simulink model "ClimaCmftStMgr"  (R2022b)
settings: Solver=FixedStepDiscrete  StartTime=0.0  StopTime=10.0  FixedStep=0.1  RelTol=1e-3
(part 2 of 2)

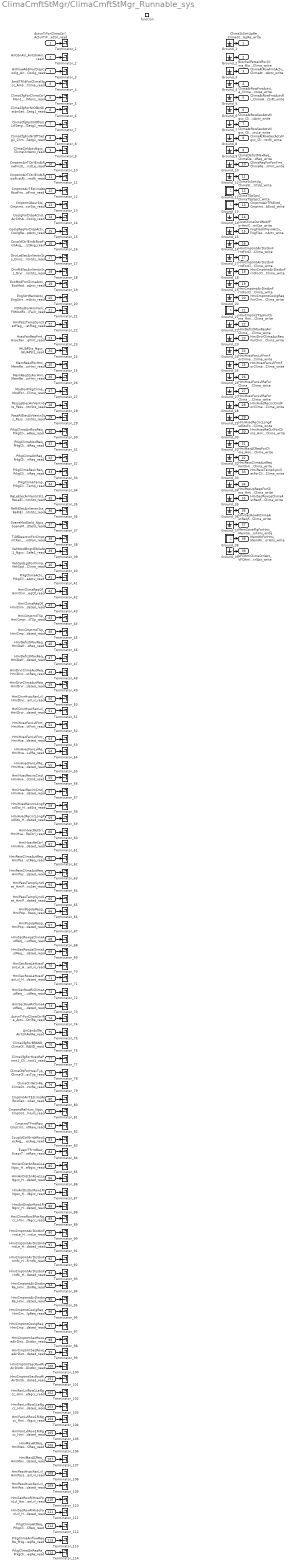
== system ClimaCmftStMgr/ClimaCmftStMgr_Runnable_sys ==
  ActvnTiForClimaCtrl_ActvnTiForClimaCtrl_read : Inport
  AirCdnAvl_AirCdnAvl_read : Inport  Port=2
  AirFlowAddlForDispCoolg_AirFlowAddlForDispCoolg_read : Inport  Port=3
  AmbTFildForClimaNgcc_AmbTFildForClima_read : Inport  Port=4
  ClimaCfgForClimaCtrlMan1_ClimaCfgForClimaCtrlMan1_read : Inport  Port=5
  ClimaCfgForNrOfAirDistbnSetg1_ClimaCfgForNrOfAirDistbnSetg1_read : Inport  Port=6
  ClimaCfgForNrOfFanLvlSetg1_ClimaCfgForNrOfFanLvlSetg1_read : Inport  Port=7
  ClimaCfgForNrOfTSetg1_ClimaCfgForNrOfTSetg1_read : Inport  Port=8
  ClimaCtrlActvNgcc_ClimaCtrlActv_read : Inport  Port=9
  CmptmtAirTCtrlErrAtRowFirstLe_CmptmtAirTCtrlErrAtRowFirstLe_read : Inport  Port=10
  CmptmtAirTCtrlErrAtRowFirstRi_CmptmtAirTCtrlErrAtRowFirstRi_read : Inport  Port=11
  CmptmtAirTEstimdAtRowFirst_CmptmtAirTEstimdAtRowFirst_read : Inport  Port=12
  CmptmtObsvrSts_CmptmtObsvrSts_read : Inport  Port=13
  CoolgForDispActvd_AirCdnActvdForDispCoolg_read : Inport  Port=14
  CoolgReqForDispActv_CoolgReqForDispActv_read : Inport  Port=15
  CoupldCtrlErrAtRowFirstAvg_CoupldCtrlErrAtRowFirstAvg_read : Inport  Port=16
  DrvrLeElecAirVentnSts_DrvrLeElecAirVentnSts_read : Inport  Port=17
  DrvrRiElecAirVentnSts_DrvrRiElecAirVentnSts_read : Inport  Port=18
  EcoModForClimaActv_EcoModForClimaActv_read : Inport  Port=19
  EngStrtRemActv_EngStrtRemActv_read : Inport  Port=20
  FltModForHmiFailr_FltModForHmiFailr_read : Inport  Port=21
  HmiPassTempSyncSetFlag_HmiPassTempSyncSetFlag_read : Inport  Port=22
  HvacFanReqFrnt_HvacFanReqFrnt_read : Inport  Port=23
  IHUAPSts_Ngcc_IHUAPSts_read : Inport  Port=24
  MemReadForHmi_MemReadForHmi_read : Inport  Port=25
  MemReqStsForHmi_MemReqStsForHmi_read : Inport  Port=26
  ModForPrkgClima_ModForPrkgClima_read : Inport  Port=27
  PassLeElecAirVentnSts_PassLeElecAirVentnSts_read : Inport  Port=28
  PassRiElecAirVentnSts_PassRiElecAirVentnSts_read : Inport  Port=29
  PrkgClimaAirflowReq_PrkgClimaAirflowReq_read : Inport  Port=30
  PrkgClimaModReq_PrkgClimaModReq_read : Inport  Port=31
  PrkgClimaOnReq_PrkgClimaOnReq_read : Inport  Port=32
  PrkgClimaRecirReq_PrkgClimaRecirReq_read : Inport  Port=33
  PrkgClimaTemp_PrkgClimaTemp_read : Inport  Port=34
  ReLeElecAirVentnSts_ReLeElecAirVentnSts_read : Inport  Port=35
  ReRiElecAirVentnSts_ReRiElecAirVentnSts_read : Inport  Port=36
  SceneModSeld_Ngcc_SceneModSeld_read : Inport  Port=37
  TiOfDeactvnForCmptmtFan_TiOfDeactvnForCmptmtFan_read : Inport  Port=38
  VehModMngtGlbSafe1_Ngcc_VehModMngtGlbSafe1_read : Inport  Port=39
  VehSpdLgtForClima_VehSpdLgtForClima_read : Inport  Port=40
  PrkgClimaActv_PrkgClimaActv_read : Inport  Port=41
  HmiClimaReqQf_HmiClimaReqQf_read : Inport  Port=42
  HmiClimaReqQf_HmiClimaReqQf_IsUpdated_read : Inport  Port=43
  HmiCmptmtTSp_HmiCmptmtTSp_read : Inport  Port=44
  HmiCmptmtTSp_HmiCmptmtTSp_IsUpdated_read : Inport  Port=45
  HmiDefrstMaxReq_HmiDefrstMaxReq_read : Inport  Port=46
  HmiDefrstMaxReq_HmiDefrstMaxReq_IsUpdated_read : Inport  Port=47
  HmiDrvrClimaAutReq_HmiDrvrClimaAutReq_read : Inport  Port=48
  HmiDrvrClimaAutReq_HmiDrvrClimaAutReq_IsUpdated_read : Inport  Port=49
  HmiDrvrHvacFanLvl_HmiDrvrHvacFanLvl_read : Inport  Port=50
  HmiDrvrHvacFanLvl_HmiDrvrHvacFanLvl_IsUpdated_read : Inport  Port=51
  HmiHvacFanLvlFrnt_HmiHvacFanLvlFrnt_read : Inport  Port=52
  HmiHvacFanLvlFrnt_HmiHvacFanLvlFrnt_IsUpdated_read : Inport  Port=53
  HmiHvacFanLvlRe_HmiHvacFanLvlRe_read : Inport  Port=54
  HmiHvacFanLvlRe_HmiHvacFanLvlRe_IsUpdated_read : Inport  Port=55
  HmiHvacRecircCmd_HmiHvacRecircCmd_read : Inport  Port=56
  HmiHvacRecircCmd_HmiHvacRecircCmd_IsUpdated_read : Inport  Port=57
  HmiHvacRecircLongPsdSts_HmiHvacRecircLongPsdSts_read : Inport  Port=58
  HmiHvacRecircLongPsdSts_HmiHvacRecircLongPsdSts_IsUpdated_read : Inport  Port=59
  HmiHvacReCtrl_HmiHvacReCtrl_read : Inport  Port=60
  HmiHvacReCtrl_HmiHvacReCtrl_IsUpdated_read : Inport  Port=61
  HmiPassClimaAutReq_HmiPassClimaAutReq_read : Inport  Port=62
  HmiPassClimaAutReq_HmiPassClimaAutReq_IsUpdated_read : Inport  Port=63
  HmiPassTempSyncSet_HmiPassTempSyncSet_read : Inport  Port=64
  HmiPassTempSyncSet_HmiPassTempSyncSet_IsUpdated_read : Inport  Port=65
  HmiPopUpResp_HmiPopUpResp_read : Inport  Port=66
  HmiPopUpResp_HmiPopUpResp_IsUpdated_read : Inport  Port=67
  HmiSecRowLeClimaAutReq_HmiSecRowLeClimaAutReq_read : Inport  Port=68
  HmiSecRowLeClimaAutReq_HmiSecRowLeClimaAutReq_IsUpdated_read : Inport  Port=69
  HmiSecRowLeHvacFanLvl_HmiSecRowLeHvacFanLvl_read : Inport  Port=70
  HmiSecRowLeHvacFanLvl_HmiSecRowLeHvacFanLvl_IsUpdated_read : Inport  Port=71
  HmiSecRowRiClimaAutReq_HmiSecRowRiClimaAutReq_read : Inport  Port=72
  HmiSecRowRiClimaAutReq_HmiSecRowRiClimaAutReq_IsUpdated_read : Inport  Port=73
  ActvnTiForClimaCtrlRe_ActvnTiForClimaCtrlRe_read : Inport  Port=74
  AirCdnAvlRe_AirCdnAvlRe_read : Inport  Port=75
  ClimaCfgForBRAND_ClimaCfgForBRAND_read : Inport  Port=76
  ClimaCfgForHvacRePrsnt1_ClimaCfgForHvacRePrsnt1_read : Inport  Port=77
  ClimaCfgForHvacTyp_ClimaCfgForHvacTyp_read : Inport  Port=78
  ClimaCtrlActvRe_ClimaCtrlActvRe_read : Inport  Port=79
  CmptmtAirTEstimdAtRowSec_CmptmtAirTEstimdAtRowSec_read : Inport  Port=80
  CmptmtRelHum_Ngcc_CmptmtRelHum_read : Inport  Port=81
  CmptmtTFrntRaw_CmptmtTFrntRaw_read : Inport  Port=82
  CoupldCtrlErrAtRowSecAvg_CoupldCtrlErrAtRowSecAvg_read : Inport  Port=83
  EvaprTFrntRaw_EvaprTFrntRaw_read : Inport  Port=84
  HmiAirDistbnRow1LeNgcc_HmiAirDistbnRow1LeNgcc_read : Inport  Port=85
  HmiAirDistbnRow1LeNgcc_HmiAirDistbnRow1LeNgcc_IsUpdated_read : Inport  Port=86
  HmiAirDistbnRow1RiNgcc_HmiAirDistbnRow1RiNgcc_read : Inport  Port=87
  HmiAirDistbnRow1RiNgcc_HmiAirDistbnRow1RiNgcc_IsUpdated_read : Inport  Port=88
  HmiClimaRow3PwrNgcc_HmiClimaRow3PwrNgcc_read : Inport  Port=89
  HmiCmptmtAirDistbnFrntLe_HmiCmptmtAirDistbnFrntLe_read : Inport  Port=90
  HmiCmptmtAirDistbnFrntLe_HmiCmptmtAirDistbnFrntLe_IsUpdated_read : Inport  Port=91
  HmiCmptmtAirDistbnFrntRi_HmiCmptmtAirDistbnFrntRi_read : Inport  Port=92
  HmiCmptmtAirDistbnFrntRi_HmiCmptmtAirDistbnFrntRi_IsUpdated_read : Inport  Port=93
  HmiCmptmtAirDistbnRe_HmiCmptmtAirDistbnRe_read : Inport  Port=94
  HmiCmptmtAirDistbnRe_HmiCmptmtAirDistbnRe_IsUpdated_read : Inport  Port=95
  HmiCmptmtCoolgReq_HmiCmptmtCoolgReq_read : Inport  Port=96
  HmiCmptmtCoolgReq_HmiCmptmtCoolgReq_IsUpdated_read : Inport  Port=97
  HmiCmptmtSecRowLeAirDistbn_HmiCmptmtSecRowLeAirDistbn_read : Inport  Port=98
  HmiCmptmtSecRowLeAirDistbn_HmiCmptmtSecRowLeAirDistbn_IsUpdated_read : Inport  Port=99
  HmiCmptmtSecRowRiAirDistbn_HmiCmptmtSecRowRiAirDistbn_read : Inport  Port=100
  HmiCmptmtSecRowRiAirDistbn_HmiCmptmtSecRowRiAirDistbn_IsUpdated_read : Inport  Port=101
  HmiFanLvlRow1LeNgcc_HmiFanLvlRow1LeNgcc_read : Inport  Port=102
  HmiFanLvlRow1LeNgcc_HmiFanLvlRow1LeNgcc_IsUpdated_read : Inport  Port=103
  HmiFanLvlRow1RiNgcc_HmiFanLvlRow1RiNgcc_read : Inport  Port=104
  HmiFanLvlRow1RiNgcc_HmiFanLvlRow1RiNgcc_IsUpdated_read : Inport  Port=105
  HmiMaxACReq_HmiMaxACReq_read : Inport  Port=106
  HmiMaxACReq_HmiMaxACReq_IsUpdated_read : Inport  Port=107
  HmiPassHvacFanLvl_HmiPassHvacFanLvl_read : Inport  Port=108
  HmiPassHvacFanLvl_HmiPassHvacFanLvl_IsUpdated_read : Inport  Port=109
  HmiSecRowRiHvacFanLvl_HmiSecRowRiHvacFanLvl_read : Inport  Port=110
  HmiSecRowRiHvacFanLvl_HmiSecRowRiHvacFanLvl_IsUpdated_read : Inport  Port=111
  PrkgClimaACReq_PrkgClimaACReq_read : Inport  Port=112
  PrkgClimaAirflowReqRe_PrkgClimaAirflowReqRe_read : Inport  Port=113
  PrkgClimaOnReqRe_PrkgClimaOnReqRe_read : Inport  Port=114
  function : TriggerPort  TriggerType=function-call  VariantControl=(inherit)  SampleTimeType=periodic  SampleTime=-1
  Ground_{1..11,14..20,22..37,39} (35 alike) : Ground
  Ground_12 : Subsystem
  Ground_13 : Subsystem
  Ground_21 : Subsystem
  Ground_38 : Subsystem
  Terminator_{1..114} (114 alike) : Terminator
  ClimaStStrtUpRe_ClimaStStrtUpRe_write : Outport
      settings: EnsureOutportIsVirtual=on
  BiochalPatseldForClima_BiochalPatseldForClima_write : Outport  Port=2
      settings: EnsureOutportIsVirtual=on
  ClimaAtRowFrntActv_ClimaAtRowFrntActv_write : Outport  Port=3
      settings: EnsureOutportIsVirtual=on
  ClimaAtRowFrntActvLe_ClimaAtRowFrntActvLe_write : Outport  Port=4
      settings: EnsureOutportIsVirtual=on
  ClimaAtRowFrntActvRi_ClimaAtRowFrntActvRi_write : Outport  Port=5
      settings: EnsureOutportIsVirtual=on
  ClimaAtRowSecActvNgcc_ClimaAtRowSecActv_write : Outport  Port=6
      settings: EnsureOutportIsVirtual=on
  ClimaAtRowSecActvNgcc_ClimaAtRowSecActvLe_write : Outport  Port=7
      settings: EnsureOutportIsVirtual=on
  ClimaAtRowSecActvNgcc_ClimaAtRowSecActvRi_write : Outport  Port=8
      settings: EnsureOutportIsVirtual=on
  ClimaDefrstMaxReq_ClimaDefrstMaxReq_write : Outport  Port=9
      settings: EnsureOutportIsVirtual=on
  ClimaReqForFanFrnt_ClimaReqForFanFrnt_write : Outport  Port=10
      settings: EnsureOutportIsVirtual=on
  ClimaStStrtUp_ClimaStStrtUp_write : Outport  Port=11
      settings: EnsureOutportIsVirtual=on
  ClimaTSpSpcl_ClimaTSpSpcl_write : Outport  Port=12
      settings: EnsureOutportIsVirtual=on
  CmptmtAirTFbExtd_CmptmtAirTFbExtd_write : Outport  Port=13
      settings: EnsureOutportIsVirtual=on
  CustClimaOvrdNotifForHmiCpt_CustClimaOvrdNotifForHmiCpt_write : Outport  Port=14
      settings: EnsureOutportIsVirtual=on
  FogFlashPrevnActv_FogFlashPrevnActv_write : Outport  Port=15
      settings: EnsureOutportIsVirtual=on
  HmiCmptmtAirDistbnFrntForClima_HmiCmptmtAirDistbnFrntForClima_write : Outport  Port=16
      settings: EnsureOutportIsVirtual=on
  HmiCmptmtAirDistbnFrntForClima_HmiCmptmtAirDistbnFrntRiForClima_write : Outport  Port=17
      settings: EnsureOutportIsVirtual=on
  HmiCmptmtAirDistbnFrntForClima_HmiCmptmtAirDistbnReForClima_write : Outport  Port=18
      settings: EnsureOutportIsVirtual=on
  HmiCmptmtAirDistbnFrntForClima_HmiCmptmtAirDistbnReRiForClima_write : Outport  Port=19
      settings: EnsureOutportIsVirtual=on
  HmiCmptmtCoolgReqForClima_HmiCmptmtCoolgReqForClima_write : Outport  Port=20
      settings: EnsureOutportIsVirtual=on
  HmiCmptmtTSpForClima_HmiCmptmtTSpForClima_write : Outport  Port=21
      settings: EnsureOutportIsVirtual=on
  HmiDefrstMaxReqForClima_HmiDefrstMaxReqForClima_write : Outport  Port=22
      settings: EnsureOutportIsVirtual=on
  HmiDrvrClimaAutReqForClima_HmiDrvrClimaAutReqForClima_write : Outport  Port=23
      settings: EnsureOutportIsVirtual=on
  HmiHvacFanLvlFrntForClima_HmiHvacFanLvlFrntForClima_write : Outport  Port=24
      settings: EnsureOutportIsVirtual=on
  HmiHvacFanLvlFrntForClima_HmiHvacFanLvlFrntRiForClima_write : Outport  Port=25
      settings: EnsureOutportIsVirtual=on
  HmiHvacFanLvlReForClima_HmiHvacFanLvlReForClima_write : Outport  Port=26
      settings: EnsureOutportIsVirtual=on
  HmiHvacFanLvlReForClima_HmiHvacFanLvlReRiForClima_write : Outport  Port=27
      settings: EnsureOutportIsVirtual=on
  HmiHvacRecircCmdForClima_HmiHvacRecircCmdForClima_write : Outport  Port=28
      settings: EnsureOutportIsVirtual=on
  HmiHvacRecircLongPsdStsForClima_HmiHvacRecircLongPsdStsForClima_write : Outport  Port=29
      settings: EnsureOutportIsVirtual=on
  HmiHvacReCtrlForClima_HmiHvacReCtrlForClima_write : Outport  Port=30
      settings: EnsureOutportIsVirtual=on
  HmiMaxACReqForClima_HmiMaxACReqForClima_write : Outport  Port=31
      settings: EnsureOutportIsVirtual=on
  HmiPassClimaAutReqForClima_HmiPassClimaAutReqForClima_write : Outport  Port=32
      settings: EnsureOutportIsVirtual=on
  HmiPassTempSyncSetForClima_HmiPassTempSyncSetForClima_write : Outport  Port=33
      settings: EnsureOutportIsVirtual=on
  HmiPopUpRespForClima_HmiPopUpRespForClima_write : Outport  Port=34
      settings: EnsureOutportIsVirtual=on
  HmiSecRowLeClimaAutReqForClima_HmiSecRowLeClimaAutReqForClima_write : Outport  Port=35
      settings: EnsureOutportIsVirtual=on
  HmiSecRowRiClimaAutReqForClima_HmiSecRowRiClimaAutReqForClima_write : Outport  Port=36
      settings: EnsureOutportIsVirtual=on
  MemSaveFlgForHmi_MemSaveFlgForHmi_write : Outport  Port=37
      settings: EnsureOutportIsVirtual=on
  MemWrForHmi_MemWrForHmi_write : Outport  Port=38
      settings: EnsureOutportIsVirtual=on
  VFCHmiClimaCtrlAct_VFCHmiClimaCtrlAct_write : Outport  Port=39
      settings: EnsureOutportIsVirtual=on
  wires:
  ActvnTiForClimaCtrl_ActvnTiForClimaCtrl_read/1 -> Terminator_1/1
  AirCdnAvl_AirCdnAvl_read/1 -> Terminator_2/1
  AirFlowAddlForDispCoolg_AirFlowAddlForDispCoolg_read/1 -> Terminator_3/1
  AmbTFildForClimaNgcc_AmbTFildForClima_read/1 -> Terminator_4/1
  ClimaCfgForClimaCtrlMan1_ClimaCfgForClimaCtrlMan1_read/1 -> Terminator_5/1
  ClimaCfgForNrOfAirDistbnSetg1_ClimaCfgForNrOfAirDistbnSetg1_read/1 -> Terminator_6/1
  ClimaCfgForNrOfFanLvlSetg1_ClimaCfgForNrOfFanLvlSetg1_read/1 -> Terminator_7/1
  ClimaCfgForNrOfTSetg1_ClimaCfgForNrOfTSetg1_read/1 -> Terminator_8/1
  ClimaCtrlActvNgcc_ClimaCtrlActv_read/1 -> Terminator_9/1
  CmptmtAirTCtrlErrAtRowFirstLe_CmptmtAirTCtrlErrAtRowFirstLe_read/1 -> Terminator_10/1
  CmptmtAirTCtrlErrAtRowFirstRi_CmptmtAirTCtrlErrAtRowFirstRi_read/1 -> Terminator_11/1
  CmptmtAirTEstimdAtRowFirst_CmptmtAirTEstimdAtRowFirst_read/1 -> Terminator_12/1
  CmptmtObsvrSts_CmptmtObsvrSts_read/1 -> Terminator_13/1
  CoolgForDispActvd_AirCdnActvdForDispCoolg_read/1 -> Terminator_14/1
  CoolgReqForDispActv_CoolgReqForDispActv_read/1 -> Terminator_15/1
  CoupldCtrlErrAtRowFirstAvg_CoupldCtrlErrAtRowFirstAvg_read/1 -> Terminator_16/1
  DrvrLeElecAirVentnSts_DrvrLeElecAirVentnSts_read/1 -> Terminator_17/1
  DrvrRiElecAirVentnSts_DrvrRiElecAirVentnSts_read/1 -> Terminator_18/1
  EcoModForClimaActv_EcoModForClimaActv_read/1 -> Terminator_19/1
  EngStrtRemActv_EngStrtRemActv_read/1 -> Terminator_20/1
  FltModForHmiFailr_FltModForHmiFailr_read/1 -> Terminator_21/1
  HmiPassTempSyncSetFlag_HmiPassTempSyncSetFlag_read/1 -> Terminator_22/1
  HvacFanReqFrnt_HvacFanReqFrnt_read/1 -> Terminator_23/1
  IHUAPSts_Ngcc_IHUAPSts_read/1 -> Terminator_24/1
  MemReadForHmi_MemReadForHmi_read/1 -> Terminator_25/1
  MemReqStsForHmi_MemReqStsForHmi_read/1 -> Terminator_26/1
  ModForPrkgClima_ModForPrkgClima_read/1 -> Terminator_27/1
  PassLeElecAirVentnSts_PassLeElecAirVentnSts_read/1 -> Terminator_28/1
  PassRiElecAirVentnSts_PassRiElecAirVentnSts_read/1 -> Terminator_29/1
  PrkgClimaAirflowReq_PrkgClimaAirflowReq_read/1 -> Terminator_30/1
  PrkgClimaModReq_PrkgClimaModReq_read/1 -> Terminator_31/1
  PrkgClimaOnReq_PrkgClimaOnReq_read/1 -> Terminator_32/1
  PrkgClimaRecirReq_PrkgClimaRecirReq_read/1 -> Terminator_33/1
  PrkgClimaTemp_PrkgClimaTemp_read/1 -> Terminator_34/1
  ReLeElecAirVentnSts_ReLeElecAirVentnSts_read/1 -> Terminator_35/1
  ReRiElecAirVentnSts_ReRiElecAirVentnSts_read/1 -> Terminator_36/1
  SceneModSeld_Ngcc_SceneModSeld_read/1 -> Terminator_37/1
  TiOfDeactvnForCmptmtFan_TiOfDeactvnForCmptmtFan_read/1 -> Terminator_38/1
  VehModMngtGlbSafe1_Ngcc_VehModMngtGlbSafe1_read/1 -> Terminator_39/1
  VehSpdLgtForClima_VehSpdLgtForClima_read/1 -> Terminator_40/1
  PrkgClimaActv_PrkgClimaActv_read/1 -> Terminator_41/1
  HmiClimaReqQf_HmiClimaReqQf_read/1 -> Terminator_42/1
  HmiClimaReqQf_HmiClimaReqQf_IsUpdated_read/1 -> Terminator_43/1
  HmiCmptmtTSp_HmiCmptmtTSp_read/1 -> Terminator_44/1
  HmiCmptmtTSp_HmiCmptmtTSp_IsUpdated_read/1 -> Terminator_45/1
  HmiDefrstMaxReq_HmiDefrstMaxReq_read/1 -> Terminator_46/1
  HmiDefrstMaxReq_HmiDefrstMaxReq_IsUpdated_read/1 -> Terminator_47/1
  HmiDrvrClimaAutReq_HmiDrvrClimaAutReq_read/1 -> Terminator_48/1
  HmiDrvrClimaAutReq_HmiDrvrClimaAutReq_IsUpdated_read/1 -> Terminator_49/1
  HmiDrvrHvacFanLvl_HmiDrvrHvacFanLvl_read/1 -> Terminator_50/1
  HmiDrvrHvacFanLvl_HmiDrvrHvacFanLvl_IsUpdated_read/1 -> Terminator_51/1
  HmiHvacFanLvlFrnt_HmiHvacFanLvlFrnt_read/1 -> Terminator_52/1
  HmiHvacFanLvlFrnt_HmiHvacFanLvlFrnt_IsUpdated_read/1 -> Terminator_53/1
  HmiHvacFanLvlRe_HmiHvacFanLvlRe_read/1 -> Terminator_54/1
  HmiHvacFanLvlRe_HmiHvacFanLvlRe_IsUpdated_read/1 -> Terminator_55/1
  HmiHvacRecircCmd_HmiHvacRecircCmd_read/1 -> Terminator_56/1
  HmiHvacRecircCmd_HmiHvacRecircCmd_IsUpdated_read/1 -> Terminator_57/1
  HmiHvacRecircLongPsdSts_HmiHvacRecircLongPsdSts_read/1 -> Terminator_58/1
  HmiHvacRecircLongPsdSts_HmiHvacRecircLongPsdSts_IsUpdated_read/1 -> Terminator_59/1
  HmiHvacReCtrl_HmiHvacReCtrl_read/1 -> Terminator_60/1
  HmiHvacReCtrl_HmiHvacReCtrl_IsUpdated_read/1 -> Terminator_61/1
  HmiPassClimaAutReq_HmiPassClimaAutReq_read/1 -> Terminator_62/1
  HmiPassClimaAutReq_HmiPassClimaAutReq_IsUpdated_read/1 -> Terminator_63/1
  HmiPassTempSyncSet_HmiPassTempSyncSet_read/1 -> Terminator_64/1
  HmiPassTempSyncSet_HmiPassTempSyncSet_IsUpdated_read/1 -> Terminator_65/1
  HmiPopUpResp_HmiPopUpResp_read/1 -> Terminator_66/1
  HmiPopUpResp_HmiPopUpResp_IsUpdated_read/1 -> Terminator_67/1
  HmiSecRowLeClimaAutReq_HmiSecRowLeClimaAutReq_read/1 -> Terminator_68/1
  HmiSecRowLeClimaAutReq_HmiSecRowLeClimaAutReq_IsUpdated_read/1 -> Terminator_69/1
  HmiSecRowLeHvacFanLvl_HmiSecRowLeHvacFanLvl_read/1 -> Terminator_70/1
  HmiSecRowLeHvacFanLvl_HmiSecRowLeHvacFanLvl_IsUpdated_read/1 -> Terminator_71/1
  HmiSecRowRiClimaAutReq_HmiSecRowRiClimaAutReq_read/1 -> Terminator_72/1
  HmiSecRowRiClimaAutReq_HmiSecRowRiClimaAutReq_IsUpdated_read/1 -> Terminator_73/1
  ActvnTiForClimaCtrlRe_ActvnTiForClimaCtrlRe_read/1 -> Terminator_74/1
  AirCdnAvlRe_AirCdnAvlRe_read/1 -> Terminator_75/1
  ClimaCfgForBRAND_ClimaCfgForBRAND_read/1 -> Terminator_76/1
  ClimaCfgForHvacRePrsnt1_ClimaCfgForHvacRePrsnt1_read/1 -> Terminator_77/1
  ClimaCfgForHvacTyp_ClimaCfgForHvacTyp_read/1 -> Terminator_78/1
  ClimaCtrlActvRe_ClimaCtrlActvRe_read/1 -> Terminator_79/1
  CmptmtAirTEstimdAtRowSec_CmptmtAirTEstimdAtRowSec_read/1 -> Terminator_80/1
  CmptmtRelHum_Ngcc_CmptmtRelHum_read/1 -> Terminator_81/1
  CmptmtTFrntRaw_CmptmtTFrntRaw_read/1 -> Terminator_82/1
  CoupldCtrlErrAtRowSecAvg_CoupldCtrlErrAtRowSecAvg_read/1 -> Terminator_83/1
  EvaprTFrntRaw_EvaprTFrntRaw_read/1 -> Terminator_84/1
  HmiAirDistbnRow1LeNgcc_HmiAirDistbnRow1LeNgcc_read/1 -> Terminator_85/1
  HmiAirDistbnRow1LeNgcc_HmiAirDistbnRow1LeNgcc_IsUpdated_read/1 -> Terminator_86/1
  HmiAirDistbnRow1RiNgcc_HmiAirDistbnRow1RiNgcc_read/1 -> Terminator_87/1
  HmiAirDistbnRow1RiNgcc_HmiAirDistbnRow1RiNgcc_IsUpdated_read/1 -> Terminator_88/1
  HmiClimaRow3PwrNgcc_HmiClimaRow3PwrNgcc_read/1 -> Terminator_89/1
  HmiCmptmtAirDistbnFrntLe_HmiCmptmtAirDistbnFrntLe_read/1 -> Terminator_90/1
  HmiCmptmtAirDistbnFrntLe_HmiCmptmtAirDistbnFrntLe_IsUpdated_read/1 -> Terminator_91/1
  HmiCmptmtAirDistbnFrntRi_HmiCmptmtAirDistbnFrntRi_read/1 -> Terminator_92/1
  HmiCmptmtAirDistbnFrntRi_HmiCmptmtAirDistbnFrntRi_IsUpdated_read/1 -> Terminator_93/1
  HmiCmptmtAirDistbnRe_HmiCmptmtAirDistbnRe_read/1 -> Terminator_94/1
  HmiCmptmtAirDistbnRe_HmiCmptmtAirDistbnRe_IsUpdated_read/1 -> Terminator_95/1
  HmiCmptmtCoolgReq_HmiCmptmtCoolgReq_read/1 -> Terminator_96/1
  HmiCmptmtCoolgReq_HmiCmptmtCoolgReq_IsUpdated_read/1 -> Terminator_97/1
  HmiCmptmtSecRowLeAirDistbn_HmiCmptmtSecRowLeAirDistbn_read/1 -> Terminator_98/1
  HmiCmptmtSecRowLeAirDistbn_HmiCmptmtSecRowLeAirDistbn_IsUpdated_read/1 -> Terminator_99/1
  HmiCmptmtSecRowRiAirDistbn_HmiCmptmtSecRowRiAirDistbn_read/1 -> Terminator_100/1
  HmiCmptmtSecRowRiAirDistbn_HmiCmptmtSecRowRiAirDistbn_IsUpdated_read/1 -> Terminator_101/1
  HmiFanLvlRow1LeNgcc_HmiFanLvlRow1LeNgcc_read/1 -> Terminator_102/1
  HmiFanLvlRow1LeNgcc_HmiFanLvlRow1LeNgcc_IsUpdated_read/1 -> Terminator_103/1
  HmiFanLvlRow1RiNgcc_HmiFanLvlRow1RiNgcc_read/1 -> Terminator_104/1
  HmiFanLvlRow1RiNgcc_HmiFanLvlRow1RiNgcc_IsUpdated_read/1 -> Terminator_105/1
  HmiMaxACReq_HmiMaxACReq_read/1 -> Terminator_106/1
  HmiMaxACReq_HmiMaxACReq_IsUpdated_read/1 -> Terminator_107/1
  HmiPassHvacFanLvl_HmiPassHvacFanLvl_read/1 -> Terminator_108/1
  HmiPassHvacFanLvl_HmiPassHvacFanLvl_IsUpdated_read/1 -> Terminator_109/1
  HmiSecRowRiHvacFanLvl_HmiSecRowRiHvacFanLvl_read/1 -> Terminator_110/1
  HmiSecRowRiHvacFanLvl_HmiSecRowRiHvacFanLvl_IsUpdated_read/1 -> Terminator_111/1
  PrkgClimaACReq_PrkgClimaACReq_read/1 -> Terminator_112/1
  PrkgClimaAirflowReqRe_PrkgClimaAirflowReqRe_read/1 -> Terminator_113/1
  PrkgClimaOnReqRe_PrkgClimaOnReqRe_read/1 -> Terminator_114/1
  Ground_1/1 -> ClimaStStrtUpRe_ClimaStStrtUpRe_write/1
  Ground_2/1 -> BiochalPatseldForClima_BiochalPatseldForClima_write/1
  Ground_3/1 -> ClimaAtRowFrntActv_ClimaAtRowFrntActv_write/1
  Ground_4/1 -> ClimaAtRowFrntActvLe_ClimaAtRowFrntActvLe_write/1
  Ground_5/1 -> ClimaAtRowFrntActvRi_ClimaAtRowFrntActvRi_write/1
  Ground_6/1 -> ClimaAtRowSecActvNgcc_ClimaAtRowSecActv_write/1
  Ground_7/1 -> ClimaAtRowSecActvNgcc_ClimaAtRowSecActvLe_write/1
  Ground_8/1 -> ClimaAtRowSecActvNgcc_ClimaAtRowSecActvRi_write/1
  Ground_9/1 -> ClimaDefrstMaxReq_ClimaDefrstMaxReq_write/1
  Ground_10/1 -> ClimaReqForFanFrnt_ClimaReqForFanFrnt_write/1
  Ground_11/1 -> ClimaStStrtUp_ClimaStStrtUp_write/1
  Ground_12/1(Out1) -> ClimaTSpSpcl_ClimaTSpSpcl_write/1
  Ground_13/1(Out1) -> CmptmtAirTFbExtd_CmptmtAirTFbExtd_write/1
  Ground_14/1 -> CustClimaOvrdNotifForHmiCpt_CustClimaOvrdNotifForHmiCpt_write/1
  Ground_15/1 -> FogFlashPrevnActv_FogFlashPrevnActv_write/1
  Ground_16/1 -> HmiCmptmtAirDistbnFrntForClima_HmiCmptmtAirDistbnFrntForClima_write/1
  Ground_17/1 -> HmiCmptmtAirDistbnFrntForClima_HmiCmptmtAirDistbnFrntRiForClima_write/1
  Ground_18/1 -> HmiCmptmtAirDistbnFrntForClima_HmiCmptmtAirDistbnReForClima_write/1
  Ground_19/1 -> HmiCmptmtAirDistbnFrntForClima_HmiCmptmtAirDistbnReRiForClima_write/1
  Ground_20/1 -> HmiCmptmtCoolgReqForClima_HmiCmptmtCoolgReqForClima_write/1
  Ground_21/1(Out1) -> HmiCmptmtTSpForClima_HmiCmptmtTSpForClima_write/1
  Ground_22/1 -> HmiDefrstMaxReqForClima_HmiDefrstMaxReqForClima_write/1
  Ground_23/1 -> HmiDrvrClimaAutReqForClima_HmiDrvrClimaAutReqForClima_write/1
  Ground_24/1 -> HmiHvacFanLvlFrntForClima_HmiHvacFanLvlFrntForClima_write/1
  Ground_25/1 -> HmiHvacFanLvlFrntForClima_HmiHvacFanLvlFrntRiForClima_write/1
  Ground_26/1 -> HmiHvacFanLvlReForClima_HmiHvacFanLvlReForClima_write/1
  Ground_27/1 -> HmiHvacFanLvlReForClima_HmiHvacFanLvlReRiForClima_write/1
  Ground_28/1 -> HmiHvacRecircCmdForClima_HmiHvacRecircCmdForClima_write/1
  Ground_29/1 -> HmiHvacRecircLongPsdStsForClima_HmiHvacRecircLongPsdStsForClima_write/1
  Ground_30/1 -> HmiHvacReCtrlForClima_HmiHvacReCtrlForClima_write/1
  Ground_31/1 -> HmiMaxACReqForClima_HmiMaxACReqForClima_write/1
  Ground_32/1 -> HmiPassClimaAutReqForClima_HmiPassClimaAutReqForClima_write/1
  Ground_33/1 -> HmiPassTempSyncSetForClima_HmiPassTempSyncSetForClima_write/1
  Ground_34/1 -> HmiPopUpRespForClima_HmiPopUpRespForClima_write/1
  Ground_35/1 -> HmiSecRowLeClimaAutReqForClima_HmiSecRowLeClimaAutReqForClima_write/1
  Ground_36/1 -> HmiSecRowRiClimaAutReqForClima_HmiSecRowRiClimaAutReqForClima_write/1
  Ground_37/1 -> MemSaveFlgForHmi_MemSaveFlgForHmi_write/1
  Ground_38/1(Out1) -> MemWrForHmi_MemWrForHmi_write/1
  Ground_39/1 -> VFCHmiClimaCtrlAct_VFCHmiClimaCtrlAct_write/1

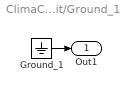
== system ClimaCmftStMgr/ClimaCmftStMgr_Init/Ground_1 ==
  Ground_1 : Ground
  Out1 : Outport  OutDataTypeStr=Bus: CmptmtAirTEstimdExtd  PortDimensions=1
  wires:
  Ground_1/1 -> Out1/1

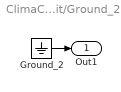
== system ClimaCmftStMgr/ClimaCmftStMgr_Init/Ground_2 ==
  Ground_2 : Ground
  Out1 : Outport  OutDataTypeStr=Bus: HmiCmptmtTSp  PortDimensions=1
  wires:
  Ground_2/1 -> Out1/1

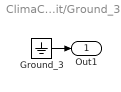
== system ClimaCmftStMgr/ClimaCmftStMgr_Init/Ground_3 ==
  Ground_3 : Ground
  Out1 : Outport  OutDataTypeStr=Bus: MemRecForHmi  PortDimensions=1
  wires:
  Ground_3/1 -> Out1/1

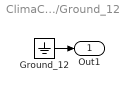
== system ClimaCmftStMgr/ClimaCmftStMgr_Runnable_sys/Ground_12 ==
  Ground_12 : Ground
  Out1 : Outport  OutDataTypeStr=Bus: ClimaTSpSpcl  PortDimensions=1
  wires:
  Ground_12/1 -> Out1/1

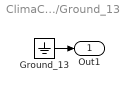
== system ClimaCmftStMgr/ClimaCmftStMgr_Runnable_sys/Ground_13 ==
  Ground_13 : Ground
  Out1 : Outport  OutDataTypeStr=Bus: CmptmtAirTEstimdExtd  PortDimensions=1
  wires:
  Ground_13/1 -> Out1/1

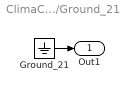
== system ClimaCmftStMgr/ClimaCmftStMgr_Runnable_sys/Ground_21 ==
  Ground_21 : Ground
  Out1 : Outport  OutDataTypeStr=Bus: HmiCmptmtTSp  PortDimensions=1
  wires:
  Ground_21/1 -> Out1/1

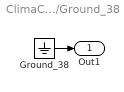
== system ClimaCmftStMgr/ClimaCmftStMgr_Runnable_sys/Ground_38 ==
  Ground_38 : Ground
  Out1 : Outport  OutDataTypeStr=Bus: MemRecForHmi  PortDimensions=1
  wires:
  Ground_38/1 -> Out1/1
